simulink model "CCMPowerMode"  (R2022b)
settings: Solver=FixedStepDiscrete  StartTime=0.0  StopTime=10.0  FixedStep=0.05  RelTol=1e-3
model workspace (MAT data in the model file):
  NvMBlockPowerModeCntData = Simulink.Signal
  NvMBlockPowerModeCntData.DataType = 'UInt8'
  NvMBlockPowerModeCntData.Dimensions = [14 1]
  NvMBlockPowerModeCntData.DimensionsMode = 'auto'
  NvMBlockPowerModeCntData.Complexity = 'real'
  NvMBlockPowerModeCntData.SampleTime = -1
  NvMBlockPowerModeCntData.SamplingMode = 'auto'
  NvMBlockPowerModeCntData.InitialValue = 'NvMBlockPowerModeCntDataInit'
  NvMBlockPowerModeCntDataInit = Simulink.Parameter
  NvMBlockPowerModeCntDataInit.Value = [0; 0; 0; 0; 0; 0; 0; 0; 0; 0; 0; 0; 0; 0]
  NvMBlockPowerModeCntDataInit.DataType = 'UInt8'
  NvMBlockPowerModeCntDataInit.Complexity = 'real'
  NvMBlockPowerModeCntDataInit.Dimensions = [14 1]
undefined symbols (not defined in the file or in its folder): HFAChlType, NvMBlockId, P_Std_ReturnType, VGAChlType
settings Model: Creator=blue.ge  LastModifiedBy=blue.ge
settings DataIOCC: SaveFormat=StructureWithTime
settings OptimizationCC: DefaultParameterBehavior=Inlined  EnhancedBackFolding=on  PassReuseOutputArgsAs=Individual arguments  ZeroExternalMemoryAtStartup=off  ZeroInternalMemoryAtStartup=off  OptimizeBlockOrder=speed  DifferentSizesBufferReuse=on  OptimizationCustomize=off  EfficientTunableParamExpr=on
settings DebuggingCC: ParameterTunabilityLossMsg=error
settings ModelReferenceCC: ModelReferenceNumInstancesAllowed=Single
settings SFSimCC: MATLABDynamicMemAlloc=off
settings RTWCC: Description=AUTOSAR  SystemTargetFile=autosar.tlc  GenCodeOnly=on  TemplateMakefile=ert_default_tmf
settings CodeAppCC: ForceParamTrailComments=on  IgnoreCustomStorageClasses=off  OperatorAnnotations=on  SimulinkDataObjDesc=on  SFDataObjDesc=on  InsertBlockDesc=on
settings STFCustomTargetCC: TargetLangStandard=C99 (ISO)  MultiwordLength=256  ParMdlRefBuildCompliant=on  CompOptLevelCompliant=on  IncludeMdlTerminateFcn=off  CombineOutputUpdateFcns=on  SuppressErrorStatus=on  ERTCustomFileBanners=on  SupportNonFinite=off  SupportComplex=off  SupportContinuousTime=off  SupportNonInlinedSFcns=off  RemoveResetFunc=on  MATLABClassNameForMDSCustomization=Simulink.SoftwareTarget.ERTCustomization  AutosarCompliant=on  UseToolchainInfoCompliant=on  LookupTableObjectStructAxisOrder=2,1,3,4,...  LUTObjectStructOrderEvenSpacing=Size,Table,Breakpoints  SystemTargetFile=autosar.tlc
settings ERTTargetCC: TargetLangStandard=C99 (ISO)  ConcurrentExecutionCompliant=off  IncludeMdlTerminateFcn=off  SuppressErrorStatus=on  SupportNonFinite=off  SupportComplex=off  ModelStepFunctionPrototypeControlCompliant=off  CPPClassGenCompliant=off  AutosarCompliant=on  LookupTableObjectStructAxisOrder=2,1,3,4,...  LUTObjectStructOrderEvenSpacing=Size,Table,Breakpoints  InlinedParameterPlacement=NonHierarchical  ERTCustomFileTemplate=example_file_process.tlc

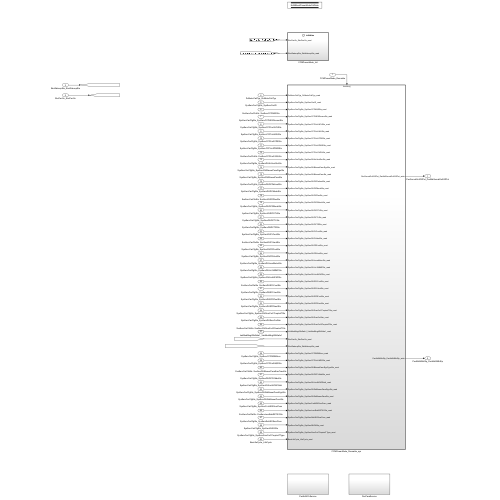
[thumbnail: Simulink's stored preview of the top-level diagram]
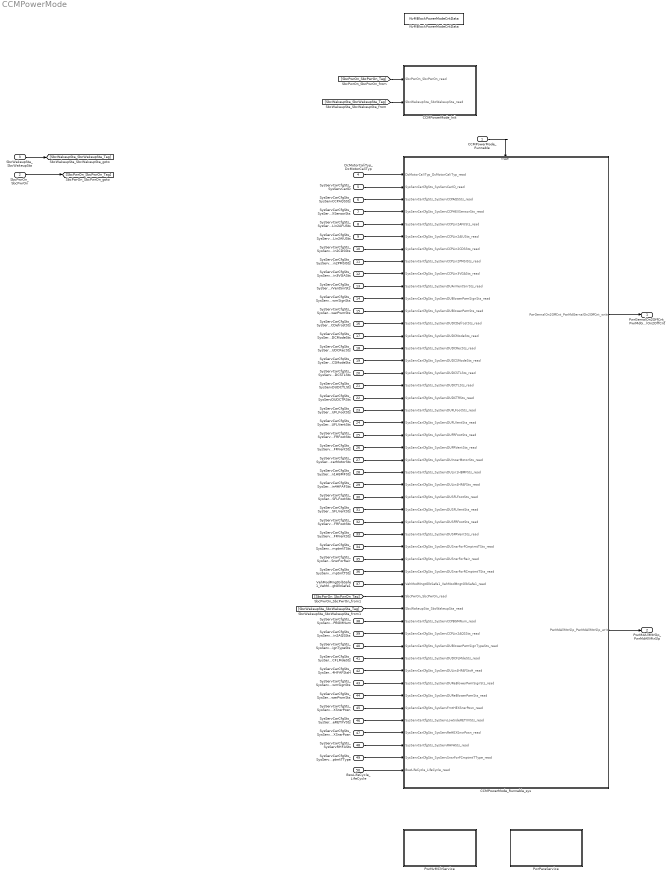
== system CCMPowerMode ==
  CCMPowerMode_Runnable : Inport  SampleTime=0.05  OutputFunctionCall=on
  SbcPwrOn_SbcPwrOn : Inport  Port=2  OutDataTypeStr=PwrOnff  PortDimensions=1
  SbcWakeupSta_SbcWakeupSta : Inport  Port=3  OutDataTypeStr=Enum: SbcWakeupSta  PortDimensions=1
  DcMotorCaliTyp_DcMotorCaliTyp : Inport  Port=4  OutDataTypeStr=UInt8  PortDimensions=1
  SysServCarCfgSts_SysServCarID : Inport  Port=5  OutDataTypeStr=Enum: SysServCarID  PortDimensions=1
  SysServCarCfgSts_SysServCCPAQSSts : Inport  Port=6  OutDataTypeStr=UInt8  PortDimensions=1
  SysServCarCfgSts_SysServCCPHEXSensorSts : Inport  Port=7  OutDataTypeStr=UInt8  PortDimensions=1
  SysServCarCfgSts_SysServCCPLin2AFUSts : Inport  Port=8  OutDataTypeStr=Boolean  PortDimensions=1
  SysServCarCfgSts_SysServCCPLin2AIUSts : Inport  Port=9  OutDataTypeStr=UInt8  PortDimensions=1
  SysServCarCfgSts_SysServCCPLin2CDSSts : Inport  Port=10  OutDataTypeStr=Boolean  PortDimensions=1
  SysServCarCfgSts_SysServCCPLin2PMSISts : Inport  Port=11  OutDataTypeStr=Boolean  PortDimensions=1
  SysServCarCfgSts_SysServCCPLin3VGASts : Inport  Port=12  OutDataTypeStr=Boolean  PortDimensions=1
  SysServCarCfgSts_SysServDUAirVentSnrSts : Inport  Port=13  OutDataTypeStr=Boolean  PortDimensions=1
  SysServCarCfgSts_SysServDUBlowerPwmSignSts : Inport  Port=14  OutDataTypeStr=Boolean  PortDimensions=1
  SysServCarCfgSts_SysServDUBlowerPwmSts : Inport  Port=15  OutDataTypeStr=Boolean  PortDimensions=1
  SysServCarCfgSts_SysServDUDCDefrostSts : Inport  Port=16  OutDataTypeStr=UInt8  PortDimensions=1
  SysServCarCfgSts_SysServDUDCModeSts : Inport  Port=17  OutDataTypeStr=UInt8  PortDimensions=1
  SysServCarCfgSts_SysServDUDCRecSts : Inport  Port=18  OutDataTypeStr=UInt8  PortDimensions=1
  SysServCarCfgSts_SysServDUDCSModeSts : Inport  Port=19  OutDataTypeStr=UInt8  PortDimensions=1
  SysServCarCfgSts_SysServDUDCSTLSts : Inport  Port=20  OutDataTypeStr=UInt8  PortDimensions=1
  SysServCarCfgSts_SysServDUDCTLSts : Inport  Port=21  OutDataTypeStr=UInt8  PortDimensions=1
  SysServCarCfgSts_SysServDUDCTRSts : Inport  Port=22  OutDataTypeStr=UInt8  PortDimensions=1
  SysServCarCfgSts_SysServDUFLFootSts : Inport  Port=23  OutDataTypeStr=UInt8  PortDimensions=1
  SysServCarCfgSts_SysServDUFLVentSts : Inport  Port=24  OutDataTypeStr=UInt8  PortDimensions=1
  SysServCarCfgSts_SysServDUFRFootSts : Inport  Port=25  OutDataTypeStr=UInt8  PortDimensions=1
  SysServCarCfgSts_SysServDUFRVentSts : Inport  Port=26  OutDataTypeStr=UInt8  PortDimensions=1
  SysServCarCfgSts_SysServDUIncarMotorSts : Inport  Port=27  OutDataTypeStr=Boolean  PortDimensions=1
  SysServCarCfgSts_SysServDULin1HBMFSts : Inport  Port=28  OutDataTypeStr=Boolean  PortDimensions=1
  SysServCarCfgSts_SysServDULin4HFAFSts : Inport  Port=29  OutDataTypeStr=UInt32  PortDimensions=1
  SysServCarCfgSts_SysServDUSFLFootSts : Inport  Port=30  OutDataTypeStr=UInt8  PortDimensions=1
  SysServCarCfgSts_SysServDUSFLVentSts : Inport  Port=31  OutDataTypeStr=UInt8  PortDimensions=1
  SysServCarCfgSts_SysServDUSFRFootSts : Inport  Port=32  OutDataTypeStr=UInt8  PortDimensions=1
  SysServCarCfgSts_SysServDUSFRVentSts : Inport  Port=33  OutDataTypeStr=UInt8  PortDimensions=1
  SysServCarCfgSts_SysServDUSnsrForFCmptmtTSts : Inport  Port=34  OutDataTypeStr=UInt8  PortDimensions=1
  SysServCarCfgSts_SysServDUSnsrForRair : Inport  Port=35  OutDataTypeStr=UInt8  PortDimensions=1
  SysServCarCfgSts_SysServDUSnsrForRCmptmtTSts : Inport  Port=36  OutDataTypeStr=UInt8  PortDimensions=1
  VehModMngtGlbSafe1_VehModMngtGlbSafe1 : Inport  Port=37  OutDataTypeStr=Bus: VehModMngtGlbSafe1  PortDimensions=1  BusOutputAsStruct=on
  SysServCarCfgSts_SysServCCPBGMNum : Inport  Port=38  OutDataTypeStr=UInt8  PortDimensions=1
  SysServCarCfgSts_SysServCCPLin2AQSSts : Inport  Port=39  OutDataTypeStr=Boolean  PortDimensions=1
  SysServCarCfgSts_SysServDUBlowerPwmSignTypeSts : Inport  Port=40  OutDataTypeStr=UInt8  PortDimensions=1
  SysServCarCfgSts_SysServDUDCFLMdeSts : Inport  Port=41  OutDataTypeStr=UInt8  PortDimensions=1
  SysServCarCfgSts_SysServDULin4HFAFStsH : Inport  Port=42  OutDataTypeStr=UInt32  PortDimensions=1
  SysServCarCfgSts_SysServDUReBlowerPwmSignSts : Inport  Port=43  OutDataTypeStr=UInt8  PortDimensions=1
  SysServCarCfgSts_SysServDUReBlowerPwmSts : Inport  Port=44  OutDataTypeStr=UInt8  PortDimensions=1
  SysServCarCfgSts_SysServFrntHEXSnsrPosn : Inport  Port=45  OutDataTypeStr=UInt8  PortDimensions=1
  SysServCarCfgSts_SysServLowSideRETXVSts : Inport  Port=46  OutDataTypeStr=UInt8  PortDimensions=1
  SysServCarCfgSts_SysServReHEXSnsrPosn : Inport  Port=47  OutDataTypeStr=UInt8  PortDimensions=1
  SysServCarCfgSts_SysServRHFASts : Inport  Port=48  OutDataTypeStr=UInt8  PortDimensions=1
  SysServCarCfgSts_SysServSnsrForFCmptmtTType : Inport  Port=49  OutDataTypeStr=UInt8  PortDimensions=1
  BswLifeCycle_LifeCycle : Inport  Port=50  OutDataTypeStr=Enum: CCMLifeCycleModeGroup  PortDimensions=1
  CCMPowerMode_Init : Subsystem
  CCMPowerMode_Runnable_sys : Subsystem
  NvMBlockPowerModeCntData : DataStoreMemory  DataStoreName=NvMBlockPowerModeCntData  InitialValue=NvMBlockPowerModeCntDataInit  ShowAdditionalParam=off  Dimensions=14  StateMustResolveToSignalObject=on
  PwrNvMClrService : Subsystem
  PwrParaService : Subsystem
  PwrGernalOn2OffCnt_PwrMdGernalOn2OffCnt : Outport  OutDataTypeStr=On2OffCnt  PortDimensions=1
  PwrMdAllMtrSlp_PwrMdAllMtrSlp : Outport  Port=2  OutDataTypeStr=Enum: PwrMdAllMtrSlp  PortDimensions=1
  wires:
  DcMotorCaliTyp_DcMotorCaliTyp/1 -> CCMPowerMode_Runnable_sys/1(DcMotorCaliTyp_DcMotorCaliTyp_read)
  SysServCarCfgSts_SysServCarID/1 -> CCMPowerMode_Runnable_sys/2(SysServCarCfgSts_SysServCarID_read)
  SysServCarCfgSts_SysServCCPAQSSts/1 -> CCMPowerMode_Runnable_sys/3(SysServCarCfgSts_SysServCCPAQSSts_read)
  SysServCarCfgSts_SysServCCPHEXSensorSts/1 -> CCMPowerMode_Runnable_sys/4(SysServCarCfgSts_SysServCCPHEXSensorSts_read)
  SysServCarCfgSts_SysServCCPLin2AFUSts/1 -> CCMPowerMode_Runnable_sys/5(SysServCarCfgSts_SysServCCPLin2AFUSts_read)
  SysServCarCfgSts_SysServCCPLin2AIUSts/1 -> CCMPowerMode_Runnable_sys/6(SysServCarCfgSts_SysServCCPLin2AIUSts_read)
  SysServCarCfgSts_SysServCCPLin2CDSSts/1 -> CCMPowerMode_Runnable_sys/7(SysServCarCfgSts_SysServCCPLin2CDSSts_read)
  SysServCarCfgSts_SysServCCPLin2PMSISts/1 -> CCMPowerMode_Runnable_sys/8(SysServCarCfgSts_SysServCCPLin2PMSISts_read)
  SysServCarCfgSts_SysServCCPLin3VGASts/1 -> CCMPowerMode_Runnable_sys/9(SysServCarCfgSts_SysServCCPLin3VGASts_read)
  SysServCarCfgSts_SysServDUAirVentSnrSts/1 -> CCMPowerMode_Runnable_sys/10(SysServCarCfgSts_SysServDUAirVentSnrSts_read)
  SysServCarCfgSts_SysServDUBlowerPwmSignSts/1 -> CCMPowerMode_Runnable_sys/11(SysServCarCfgSts_SysServDUBlowerPwmSignSts_read)
  SysServCarCfgSts_SysServDUBlowerPwmSts/1 -> CCMPowerMode_Runnable_sys/12(SysServCarCfgSts_SysServDUBlowerPwmSts_read)
  SysServCarCfgSts_SysServDUDCDefrostSts/1 -> CCMPowerMode_Runnable_sys/13(SysServCarCfgSts_SysServDUDCDefrostSts_read)
  SysServCarCfgSts_SysServDUDCModeSts/1 -> CCMPowerMode_Runnable_sys/14(SysServCarCfgSts_SysServDUDCModeSts_read)
  SysServCarCfgSts_SysServDUDCRecSts/1 -> CCMPowerMode_Runnable_sys/15(SysServCarCfgSts_SysServDUDCRecSts_read)
  SysServCarCfgSts_SysServDUDCSModeSts/1 -> CCMPowerMode_Runnable_sys/16(SysServCarCfgSts_SysServDUDCSModeSts_read)
  SysServCarCfgSts_SysServDUDCSTLSts/1 -> CCMPowerMode_Runnable_sys/17(SysServCarCfgSts_SysServDUDCSTLSts_read)
  SysServCarCfgSts_SysServDUDCTLSts/1 -> CCMPowerMode_Runnable_sys/18(SysServCarCfgSts_SysServDUDCTLSts_read)
  SysServCarCfgSts_SysServDUDCTRSts/1 -> CCMPowerMode_Runnable_sys/19(SysServCarCfgSts_SysServDUDCTRSts_read)
  SysServCarCfgSts_SysServDUFLFootSts/1 -> CCMPowerMode_Runnable_sys/20(SysServCarCfgSts_SysServDUFLFootSts_read)
  SysServCarCfgSts_SysServDUFLVentSts/1 -> CCMPowerMode_Runnable_sys/21(SysServCarCfgSts_SysServDUFLVentSts_read)
  SysServCarCfgSts_SysServDUFRFootSts/1 -> CCMPowerMode_Runnable_sys/22(SysServCarCfgSts_SysServDUFRFootSts_read)
  SysServCarCfgSts_SysServDUFRVentSts/1 -> CCMPowerMode_Runnable_sys/23(SysServCarCfgSts_SysServDUFRVentSts_read)
  SysServCarCfgSts_SysServDUIncarMotorSts/1 -> CCMPowerMode_Runnable_sys/24(SysServCarCfgSts_SysServDUIncarMotorSts_read)
  SysServCarCfgSts_SysServDULin1HBMFSts/1 -> CCMPowerMode_Runnable_sys/25(SysServCarCfgSts_SysServDULin1HBMFSts_read)
  SysServCarCfgSts_SysServDULin4HFAFSts/1 -> CCMPowerMode_Runnable_sys/26(SysServCarCfgSts_SysServDULin4HFAFSts_read)
  SysServCarCfgSts_SysServDUSFLFootSts/1 -> CCMPowerMode_Runnable_sys/27(SysServCarCfgSts_SysServDUSFLFootSts_read)
  SysServCarCfgSts_SysServDUSFLVentSts/1 -> CCMPowerMode_Runnable_sys/28(SysServCarCfgSts_SysServDUSFLVentSts_read)
  SysServCarCfgSts_SysServDUSFRFootSts/1 -> CCMPowerMode_Runnable_sys/29(SysServCarCfgSts_SysServDUSFRFootSts_read)
  SysServCarCfgSts_SysServDUSFRVentSts/1 -> CCMPowerMode_Runnable_sys/30(SysServCarCfgSts_SysServDUSFRVentSts_read)
  SysServCarCfgSts_SysServDUSnsrForFCmptmtTSts/1 -> CCMPowerMode_Runnable_sys/31(SysServCarCfgSts_SysServDUSnsrForFCmptmtTSts_read)
  SysServCarCfgSts_SysServDUSnsrForRair/1 -> CCMPowerMode_Runnable_sys/32(SysServCarCfgSts_SysServDUSnsrForRair_read)
  SysServCarCfgSts_SysServDUSnsrForRCmptmtTSts/1 -> CCMPowerMode_Runnable_sys/33(SysServCarCfgSts_SysServDUSnsrForRCmptmtTSts_read)
  VehModMngtGlbSafe1_VehModMngtGlbSafe1/1 -> CCMPowerMode_Runnable_sys/34(VehModMngtGlbSafe1_VehModMngtGlbSafe1_read)
  SysServCarCfgSts_SysServCCPBGMNum/1 -> CCMPowerMode_Runnable_sys/37(SysServCarCfgSts_SysServCCPBGMNum_read)
  SysServCarCfgSts_SysServCCPLin2AQSSts/1 -> CCMPowerMode_Runnable_sys/38(SysServCarCfgSts_SysServCCPLin2AQSSts_read)
  SysServCarCfgSts_SysServDUBlowerPwmSignTypeSts/1 -> CCMPowerMode_Runnable_sys/39(SysServCarCfgSts_SysServDUBlowerPwmSignTypeSts_read)
  SysServCarCfgSts_SysServDUDCFLMdeSts/1 -> CCMPowerMode_Runnable_sys/40(SysServCarCfgSts_SysServDUDCFLMdeSts_read)
  SysServCarCfgSts_SysServDULin4HFAFStsH/1 -> CCMPowerMode_Runnable_sys/41(SysServCarCfgSts_SysServDULin4HFAFStsH_read)
  SysServCarCfgSts_SysServDUReBlowerPwmSignSts/1 -> CCMPowerMode_Runnable_sys/42(SysServCarCfgSts_SysServDUReBlowerPwmSignSts_read)
  SysServCarCfgSts_SysServDUReBlowerPwmSts/1 -> CCMPowerMode_Runnable_sys/43(SysServCarCfgSts_SysServDUReBlowerPwmSts_read)
  SysServCarCfgSts_SysServFrntHEXSnsrPosn/1 -> CCMPowerMode_Runnable_sys/44(SysServCarCfgSts_SysServFrntHEXSnsrPosn_read)
  SysServCarCfgSts_SysServLowSideRETXVSts/1 -> CCMPowerMode_Runnable_sys/45(SysServCarCfgSts_SysServLowSideRETXVSts_read)
  SysServCarCfgSts_SysServReHEXSnsrPosn/1 -> CCMPowerMode_Runnable_sys/46(SysServCarCfgSts_SysServReHEXSnsrPosn_read)
  SysServCarCfgSts_SysServRHFASts/1 -> CCMPowerMode_Runnable_sys/47(SysServCarCfgSts_SysServRHFASts_read)
  SysServCarCfgSts_SysServSnsrForFCmptmtTType/1 -> CCMPowerMode_Runnable_sys/48(SysServCarCfgSts_SysServSnsrForFCmptmtTType_read)
  CCMPowerMode_Runnable_sys/1(PwrGernalOn2OffCnt_PwrMdGernalOn2OffCnt_write) -> PwrGernalOn2OffCnt_PwrMdGernalOn2OffCnt/1
  CCMPowerMode_Runnable_sys/2(PwrMdAllMtrSlp_PwrMdAllMtrSlp_write) -> PwrMdAllMtrSlp_PwrMdAllMtrSlp/1
  BswLifeCycle_LifeCycle/1 -> CCMPowerMode_Runnable_sys/49(BswLifeCycle_LifeCycle_read)
  SbcWakeupSta_SbcWakeupSta/1 -> [SbcWakeupSta_SbcWakeupSta_Tag]
  [SbcWakeupSta_SbcWakeupSta_Tag] -> CCMPowerMode_Init/2(SbcWakeupSta_SbcWakeupSta_read)
  [SbcWakeupSta_SbcWakeupSta_Tag] -> CCMPowerMode_Runnable_sys/36(SbcWakeupSta_SbcWakeupSta_read)
  SbcPwrOn_SbcPwrOn/1 -> [SbcPwrOn_SbcPwrOn_Tag]
  [SbcPwrOn_SbcPwrOn_Tag] -> CCMPowerMode_Init/1(SbcPwrOn_SbcPwrOn_read)
  [SbcPwrOn_SbcPwrOn_Tag] -> CCMPowerMode_Runnable_sys/35(SbcPwrOn_SbcPwrOn_read)
  CCMPowerMode_Runnable/1 -> CCMPowerMode_Runnable_sys/Trigger

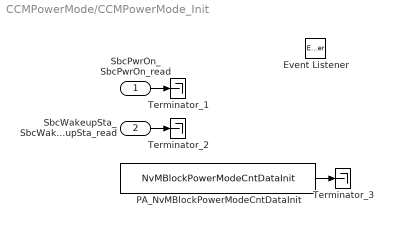
== system CCMPowerMode/CCMPowerMode_Init ==
  SbcPwrOn_SbcPwrOn_read : Inport
  SbcWakeupSta_SbcWakeupSta_read : Inport  Port=2
  Event Listener : EventListener  EventName=reset
  PA_NvMBlockPowerModeCntDataInit : Constant  Value=NvMBlockPowerModeCntDataInit
  Terminator_1 : Terminator
  Terminator_2 : Terminator
  Terminator_3 : Terminator
  wires:
  SbcPwrOn_SbcPwrOn_read/1 -> Terminator_1/1
  SbcWakeupSta_SbcWakeupSta_read/1 -> Terminator_2/1
  PA_NvMBlockPowerModeCntDataInit/1 -> Terminator_3/1

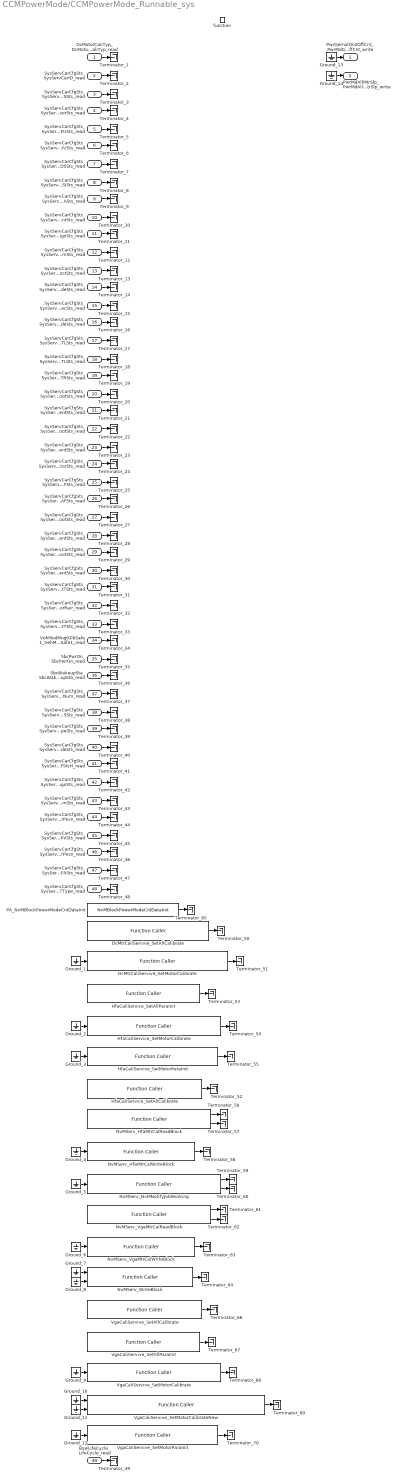
== system CCMPowerMode/CCMPowerMode_Runnable_sys ==
  DcMotorCaliTyp_DcMotorCaliTyp_read : Inport
  SysServCarCfgSts_SysServCarID_read : Inport  Port=2
  SysServCarCfgSts_SysServCCPAQSSts_read : Inport  Port=3
  SysServCarCfgSts_SysServCCPHEXSensorSts_read : Inport  Port=4
  SysServCarCfgSts_SysServCCPLin2AFUSts_read : Inport  Port=5
  SysServCarCfgSts_SysServCCPLin2AIUSts_read : Inport  Port=6
  SysServCarCfgSts_SysServCCPLin2CDSSts_read : Inport  Port=7
  SysServCarCfgSts_SysServCCPLin2PMSISts_read : Inport  Port=8
  SysServCarCfgSts_SysServCCPLin3VGASts_read : Inport  Port=9
  SysServCarCfgSts_SysServDUAirVentSnrSts_read : Inport  Port=10
  SysServCarCfgSts_SysServDUBlowerPwmSignSts_read : Inport  Port=11
  SysServCarCfgSts_SysServDUBlowerPwmSts_read : Inport  Port=12
  SysServCarCfgSts_SysServDUDCDefrostSts_read : Inport  Port=13
  SysServCarCfgSts_SysServDUDCModeSts_read : Inport  Port=14
  SysServCarCfgSts_SysServDUDCRecSts_read : Inport  Port=15
  SysServCarCfgSts_SysServDUDCSModeSts_read : Inport  Port=16
  SysServCarCfgSts_SysServDUDCSTLSts_read : Inport  Port=17
  SysServCarCfgSts_SysServDUDCTLSts_read : Inport  Port=18
  SysServCarCfgSts_SysServDUDCTRSts_read : Inport  Port=19
  SysServCarCfgSts_SysServDUFLFootSts_read : Inport  Port=20
  SysServCarCfgSts_SysServDUFLVentSts_read : Inport  Port=21
  SysServCarCfgSts_SysServDUFRFootSts_read : Inport  Port=22
  SysServCarCfgSts_SysServDUFRVentSts_read : Inport  Port=23
  SysServCarCfgSts_SysServDUIncarMotorSts_read : Inport  Port=24
  SysServCarCfgSts_SysServDULin1HBMFSts_read : Inport  Port=25
  SysServCarCfgSts_SysServDULin4HFAFSts_read : Inport  Port=26
  SysServCarCfgSts_SysServDUSFLFootSts_read : Inport  Port=27
  SysServCarCfgSts_SysServDUSFLVentSts_read : Inport  Port=28
  SysServCarCfgSts_SysServDUSFRFootSts_read : Inport  Port=29
  SysServCarCfgSts_SysServDUSFRVentSts_read : Inport  Port=30
  SysServCarCfgSts_SysServDUSnsrForFCmptmtTSts_read : Inport  Port=31
  SysServCarCfgSts_SysServDUSnsrForRair_read : Inport  Port=32
  SysServCarCfgSts_SysServDUSnsrForRCmptmtTSts_read : Inport  Port=33
  VehModMngtGlbSafe1_VehModMngtGlbSafe1_read : Inport  Port=34
  SbcPwrOn_SbcPwrOn_read : Inport  Port=35
  SbcWakeupSta_SbcWakeupSta_read : Inport  Port=36
  SysServCarCfgSts_SysServCCPBGMNum_read : Inport  Port=37
  SysServCarCfgSts_SysServCCPLin2AQSSts_read : Inport  Port=38
  SysServCarCfgSts_SysServDUBlowerPwmSignTypeSts_read : Inport  Port=39
  SysServCarCfgSts_SysServDUDCFLMdeSts_read : Inport  Port=40
  SysServCarCfgSts_SysServDULin4HFAFStsH_read : Inport  Port=41
  SysServCarCfgSts_SysServDUReBlowerPwmSignSts_read : Inport  Port=42
  SysServCarCfgSts_SysServDUReBlowerPwmSts_read : Inport  Port=43
  SysServCarCfgSts_SysServFrntHEXSnsrPosn_read : Inport  Port=44
  SysServCarCfgSts_SysServLowSideRETXVSts_read : Inport  Port=45
  SysServCarCfgSts_SysServReHEXSnsrPosn_read : Inport  Port=46
  SysServCarCfgSts_SysServRHFASts_read : Inport  Port=47
  SysServCarCfgSts_SysServSnsrForFCmptmtTType_read : Inport  Port=48
  BswLifeCycle_LifeCycle_read : Inport  Port=49
  function : TriggerPort  TriggerType=function-call  VariantControl=(inherit)  SampleTimeType=periodic  SampleTime=-1
  DcMtrCaliServive_SetAllCalibrate : FunctionCaller  FunctionPrototype=ERR = DcMtrCaliServive_SetAllCalibrate()  OutputArgumentSpecifications=P_Std_ReturnType
  DcMtrCaliServive_SetMotorCalibrate : FunctionCaller  FunctionPrototype=ERR = DcMtrCaliServive_SetMotorCalibrate(Channel)  InputArgumentSpecifications=uint8(1)  OutputArgumentSpecifications=P_Std_ReturnType
  Ground_{1..14} (14 alike) : Ground
  HfaCaliService_SetAllCalibrate : FunctionCaller  FunctionPrototype=ERR = HfaCaliService_SetAllCalibrate()  OutputArgumentSpecifications=P_Std_ReturnType
  HfaCaliService_SetAllParaInit : FunctionCaller  FunctionPrototype=ERR = HfaCaliService_SetAllParaInit()  OutputArgumentSpecifications=P_Std_ReturnType
  HfaCaliService_SetMotorCalibrate : FunctionCaller  FunctionPrototype=ERR = HfaCaliService_SetMotorCalibrate(Channel)  InputArgumentSpecifications=HFAChlType(0)  OutputArgumentSpecifications=P_Std_ReturnType
  HfaCaliService_SetMotorParaInit : FunctionCaller  FunctionPrototype=ERR = HfaCaliService_SetMotorParaInit(Channel)  InputArgumentSpecifications=HFAChlType(0)  OutputArgumentSpecifications=P_Std_ReturnType
  NvMServ_HfaMtrCalReadBlock : FunctionCaller  FunctionPrototype=[Data,ERR] = NvMServ_HfaMtrCalReadBlock()  OutputArgumentSpecifications=uint8([1]), P_Std_ReturnType
  NvMServ_HfaMtrCalWriteBlock : FunctionCaller  FunctionPrototype=ERR = NvMServ_HfaMtrCalWriteBlock(Data)  InputArgumentSpecifications=uint8([1])  OutputArgumentSpecifications=P_Std_ReturnType
  NvMServ_NvMNotifyJobWorking : FunctionCaller  FunctionPrototype=[Status,ERR] = NvMServ_NvMNotifyJobWorking(BlockId)  InputArgumentSpecifications=NvMBlockId(0)  OutputArgumentSpecifications=uint8(1), P_Std_ReturnType
  NvMServ_VgaMtrCalReadBlock : FunctionCaller  FunctionPrototype=[Data,ERR] = NvMServ_VgaMtrCalReadBlock()  OutputArgumentSpecifications=uint8([1]), P_Std_ReturnType
  NvMServ_VgaMtrCalWriteBlock : FunctionCaller  FunctionPrototype=ERR = NvMServ_VgaMtrCalWriteBlock(Data)  InputArgumentSpecifications=uint8([1])  OutputArgumentSpecifications=P_Std_ReturnType
  NvMServ_WriteBlock : FunctionCaller  FunctionPrototype=ERR = NvMServ_WriteBlock(BlockId,Data)  InputArgumentSpecifications=NvMBlockId(0), uint8([1])  OutputArgumentSpecifications=P_Std_ReturnType
  PA_NvMBlockPowerModeCntDataInit : Constant  Value=NvMBlockPowerModeCntDataInit
  Terminator_{1..70} (70 alike) : Terminator
  VgaCaliServive_SetAllCalibrate : FunctionCaller  FunctionPrototype=ERR = VgaCaliServive_SetAllCalibrate()  OutputArgumentSpecifications=P_Std_ReturnType
  VgaCaliServive_SetAllParaInit : FunctionCaller  FunctionPrototype=ERR = VgaCaliServive_SetAllParaInit()  OutputArgumentSpecifications=P_Std_ReturnType
  VgaCaliServive_SetMotorCalibrate : FunctionCaller  FunctionPrototype=ERR = VgaCaliServive_SetMotorCalibrate(Channel)  InputArgumentSpecifications=VGAChlType(0)  OutputArgumentSpecifications=P_Std_ReturnType
  VgaCaliServive_SetMotorCalibrateNew : FunctionCaller  FunctionPrototype=ERR = VgaCaliServive_SetMotorCalibrateNew(Channel,LearnType)  InputArgumentSpecifications=VGAChlType(0), uint8(1)  OutputArgumentSpecifications=P_Std_ReturnType
  VgaCaliServive_SetMotorParaInit : FunctionCaller  FunctionPrototype=ERR = VgaCaliServive_SetMotorParaInit(Channel)  InputArgumentSpecifications=VGAChlType(0)  OutputArgumentSpecifications=P_Std_ReturnType
  PwrGernalOn2OffCnt_PwrMdGernalOn2OffCnt_write : Outport
      settings: EnsureOutportIsVirtual=on
  PwrMdAllMtrSlp_PwrMdAllMtrSlp_write : Outport  Port=2
      settings: EnsureOutportIsVirtual=on
  wires:
  DcMotorCaliTyp_DcMotorCaliTyp_read/1 -> Terminator_1/1
  SysServCarCfgSts_SysServCarID_read/1 -> Terminator_2/1
  SysServCarCfgSts_SysServCCPAQSSts_read/1 -> Terminator_3/1
  SysServCarCfgSts_SysServCCPHEXSensorSts_read/1 -> Terminator_4/1
  SysServCarCfgSts_SysServCCPLin2AFUSts_read/1 -> Terminator_5/1
  SysServCarCfgSts_SysServCCPLin2AIUSts_read/1 -> Terminator_6/1
  SysServCarCfgSts_SysServCCPLin2CDSSts_read/1 -> Terminator_7/1
  SysServCarCfgSts_SysServCCPLin2PMSISts_read/1 -> Terminator_8/1
  SysServCarCfgSts_SysServCCPLin3VGASts_read/1 -> Terminator_9/1
  SysServCarCfgSts_SysServDUAirVentSnrSts_read/1 -> Terminator_10/1
  SysServCarCfgSts_SysServDUBlowerPwmSignSts_read/1 -> Terminator_11/1
  SysServCarCfgSts_SysServDUBlowerPwmSts_read/1 -> Terminator_12/1
  SysServCarCfgSts_SysServDUDCDefrostSts_read/1 -> Terminator_13/1
  SysServCarCfgSts_SysServDUDCModeSts_read/1 -> Terminator_14/1
  SysServCarCfgSts_SysServDUDCRecSts_read/1 -> Terminator_15/1
  SysServCarCfgSts_SysServDUDCSModeSts_read/1 -> Terminator_16/1
  SysServCarCfgSts_SysServDUDCSTLSts_read/1 -> Terminator_17/1
  SysServCarCfgSts_SysServDUDCTLSts_read/1 -> Terminator_18/1
  SysServCarCfgSts_SysServDUDCTRSts_read/1 -> Terminator_19/1
  SysServCarCfgSts_SysServDUFLFootSts_read/1 -> Terminator_20/1
  SysServCarCfgSts_SysServDUFLVentSts_read/1 -> Terminator_21/1
  SysServCarCfgSts_SysServDUFRFootSts_read/1 -> Terminator_22/1
  SysServCarCfgSts_SysServDUFRVentSts_read/1 -> Terminator_23/1
  SysServCarCfgSts_SysServDUIncarMotorSts_read/1 -> Terminator_24/1
  SysServCarCfgSts_SysServDULin1HBMFSts_read/1 -> Terminator_25/1
  SysServCarCfgSts_SysServDULin4HFAFSts_read/1 -> Terminator_26/1
  SysServCarCfgSts_SysServDUSFLFootSts_read/1 -> Terminator_27/1
  SysServCarCfgSts_SysServDUSFLVentSts_read/1 -> Terminator_28/1
  SysServCarCfgSts_SysServDUSFRFootSts_read/1 -> Terminator_29/1
  SysServCarCfgSts_SysServDUSFRVentSts_read/1 -> Terminator_30/1
  SysServCarCfgSts_SysServDUSnsrForFCmptmtTSts_read/1 -> Terminator_31/1
  SysServCarCfgSts_SysServDUSnsrForRair_read/1 -> Terminator_32/1
  SysServCarCfgSts_SysServDUSnsrForRCmptmtTSts_read/1 -> Terminator_33/1
  VehModMngtGlbSafe1_VehModMngtGlbSafe1_read/1 -> Terminator_34/1
  SbcPwrOn_SbcPwrOn_read/1 -> Terminator_35/1
  SbcWakeupSta_SbcWakeupSta_read/1 -> Terminator_36/1
  SysServCarCfgSts_SysServCCPBGMNum_read/1 -> Terminator_37/1
  SysServCarCfgSts_SysServCCPLin2AQSSts_read/1 -> Terminator_38/1
  SysServCarCfgSts_SysServDUBlowerPwmSignTypeSts_read/1 -> Terminator_39/1
  SysServCarCfgSts_SysServDUDCFLMdeSts_read/1 -> Terminator_40/1
  SysServCarCfgSts_SysServDULin4HFAFStsH_read/1 -> Terminator_41/1
  SysServCarCfgSts_SysServDUReBlowerPwmSignSts_read/1 -> Terminator_42/1
  SysServCarCfgSts_SysServDUReBlowerPwmSts_read/1 -> Terminator_43/1
  SysServCarCfgSts_SysServFrntHEXSnsrPosn_read/1 -> Terminator_44/1
  SysServCarCfgSts_SysServLowSideRETXVSts_read/1 -> Terminator_45/1
  SysServCarCfgSts_SysServReHEXSnsrPosn_read/1 -> Terminator_46/1
  SysServCarCfgSts_SysServRHFASts_read/1 -> Terminator_47/1
  SysServCarCfgSts_SysServSnsrForFCmptmtTType_read/1 -> Terminator_48/1
  BswLifeCycle_LifeCycle_read/1 -> Terminator_49/1
  DcMtrCaliServive_SetAllCalibrate/1 -> Terminator_50/1
  Ground_1/1 -> DcMtrCaliServive_SetMotorCalibrate/1
  DcMtrCaliServive_SetMotorCalibrate/1 -> Terminator_51/1
  HfaCaliService_SetAllCalibrate/1 -> Terminator_52/1
  HfaCaliService_SetAllParaInit/1 -> Terminator_53/1
  Ground_2/1 -> HfaCaliService_SetMotorCalibrate/1
  HfaCaliService_SetMotorCalibrate/1 -> Terminator_54/1
  Ground_3/1 -> HfaCaliService_SetMotorParaInit/1
  HfaCaliService_SetMotorParaInit/1 -> Terminator_55/1
  NvMServ_HfaMtrCalReadBlock/1 -> Terminator_56/1
  NvMServ_HfaMtrCalReadBlock/2 -> Terminator_57/1
  Ground_4/1 -> NvMServ_HfaMtrCalWriteBlock/1
  NvMServ_HfaMtrCalWriteBlock/1 -> Terminator_58/1
  Ground_5/1 -> NvMServ_NvMNotifyJobWorking/1
  NvMServ_NvMNotifyJobWorking/1 -> Terminator_59/1
  NvMServ_NvMNotifyJobWorking/2 -> Terminator_60/1
  NvMServ_VgaMtrCalReadBlock/1 -> Terminator_61/1
  NvMServ_VgaMtrCalReadBlock/2 -> Terminator_62/1
  Ground_6/1 -> NvMServ_VgaMtrCalWriteBlock/1
  NvMServ_VgaMtrCalWriteBlock/1 -> Terminator_63/1
  Ground_7/1 -> NvMServ_WriteBlock/1
  Ground_8/1 -> NvMServ_WriteBlock/2
  NvMServ_WriteBlock/1 -> Terminator_64/1
  PA_NvMBlockPowerModeCntDataInit/1 -> Terminator_65/1
  VgaCaliServive_SetAllCalibrate/1 -> Terminator_66/1
  VgaCaliServive_SetAllParaInit/1 -> Terminator_67/1
  Ground_9/1 -> VgaCaliServive_SetMotorCalibrate/1
  VgaCaliServive_SetMotorCalibrate/1 -> Terminator_68/1
  Ground_10/1 -> VgaCaliServive_SetMotorCalibrateNew/1
  Ground_11/1 -> VgaCaliServive_SetMotorCalibrateNew/2
  VgaCaliServive_SetMotorCalibrateNew/1 -> Terminator_69/1
  Ground_12/1 -> VgaCaliServive_SetMotorParaInit/1
  VgaCaliServive_SetMotorParaInit/1 -> Terminator_70/1
  Ground_13/1 -> PwrGernalOn2OffCnt_PwrMdGernalOn2OffCnt_write/1
  Ground_14/1 -> PwrMdAllMtrSlp_PwrMdAllMtrSlp_write/1

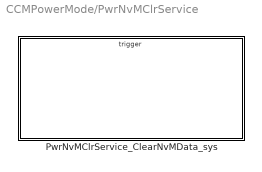
== system CCMPowerMode/PwrNvMClrService ==
  PwrNvMClrService_ClearNvMData_sys : Subsystem

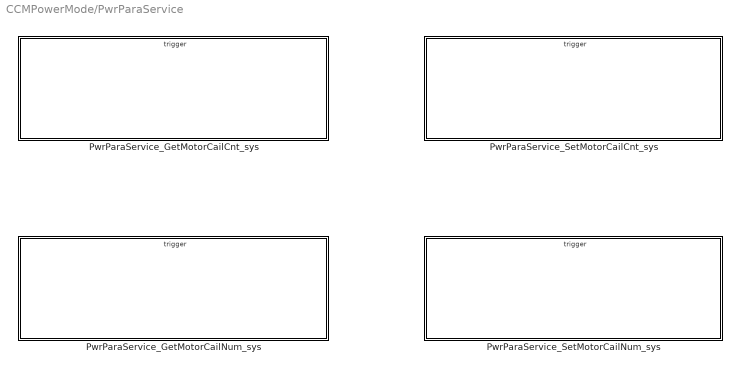
== system CCMPowerMode/PwrParaService ==
  PwrParaService_GetMotorCailCnt_sys : Subsystem
  PwrParaService_GetMotorCailNum_sys : Subsystem
  PwrParaService_SetMotorCailCnt_sys : Subsystem
  PwrParaService_SetMotorCailNum_sys : Subsystem

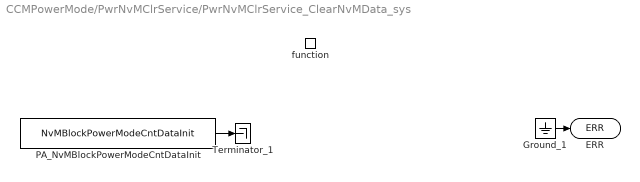
== system CCMPowerMode/PwrNvMClrService/PwrNvMClrService_ClearNvMData_sys ==
  function : TriggerPort  TriggerType=function-call  IsSimulinkFunction=on  FunctionName=PwrNvMClrService_ClearNvMData  VariantControl=(inherit)
  Ground_1 : Ground
  PA_NvMBlockPowerModeCntDataInit : Constant  Value=NvMBlockPowerModeCntDataInit
  Terminator_1 : Terminator
  ERR : ArgOut  OutDataTypeStr=Std_ReturnType  ArgumentName=ERR
  wires:
  PA_NvMBlockPowerModeCntDataInit/1 -> Terminator_1/1
  Ground_1/1 -> ERR/1

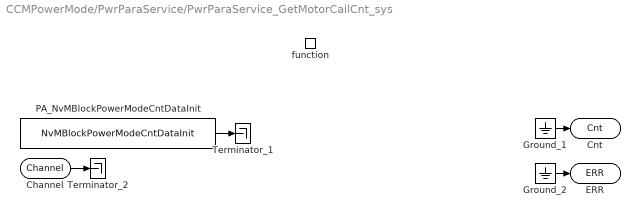
== system CCMPowerMode/PwrParaService/PwrParaService_GetMotorCailCnt_sys ==
  function : TriggerPort  TriggerType=function-call  IsSimulinkFunction=on  FunctionName=PwrParaService_GetMotorCailCnt  VariantControl=(inherit)
  Ground_1 : Ground
  Ground_2 : Ground
  PA_NvMBlockPowerModeCntDataInit : Constant  Value=NvMBlockPowerModeCntDataInit
  Terminator_1 : Terminator
  Terminator_2 : Terminator
  Channel : ArgIn  OutDataTypeStr=Enum: PowerChlType  ArgumentName=Channel
  Cnt : ArgOut  OutDataTypeStr=UInt8  ArgumentName=Cnt
  ERR : ArgOut  Port=2  OutDataTypeStr=Std_ReturnType  ArgumentName=ERR
  wires:
  PA_NvMBlockPowerModeCntDataInit/1 -> Terminator_1/1
  Channel/1 -> Terminator_2/1
  Ground_1/1 -> Cnt/1
  Ground_2/1 -> ERR/1

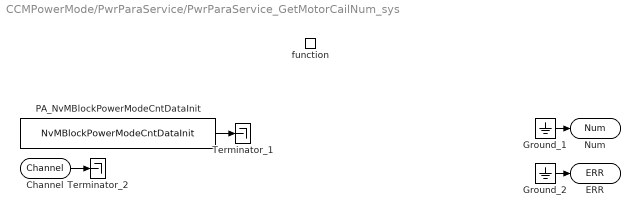
== system CCMPowerMode/PwrParaService/PwrParaService_GetMotorCailNum_sys ==
  function : TriggerPort  TriggerType=function-call  IsSimulinkFunction=on  FunctionName=PwrParaService_GetMotorCailNum  VariantControl=(inherit)
  Ground_1 : Ground
  Ground_2 : Ground
  PA_NvMBlockPowerModeCntDataInit : Constant  Value=NvMBlockPowerModeCntDataInit
  Terminator_1 : Terminator
  Terminator_2 : Terminator
  Channel : ArgIn  OutDataTypeStr=Enum: PowerChlType  ArgumentName=Channel
  Num : ArgOut  OutDataTypeStr=UInt8  ArgumentName=Num
  ERR : ArgOut  Port=2  OutDataTypeStr=Std_ReturnType  ArgumentName=ERR
  wires:
  PA_NvMBlockPowerModeCntDataInit/1 -> Terminator_1/1
  Channel/1 -> Terminator_2/1
  Ground_1/1 -> Num/1
  Ground_2/1 -> ERR/1

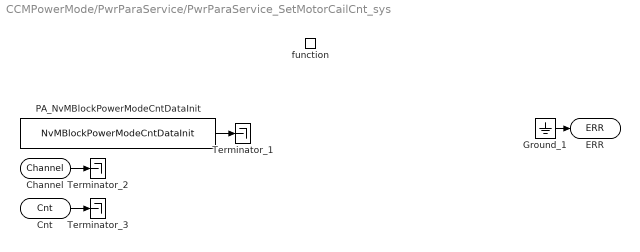
== system CCMPowerMode/PwrParaService/PwrParaService_SetMotorCailCnt_sys ==
  function : TriggerPort  TriggerType=function-call  IsSimulinkFunction=on  FunctionName=PwrParaService_SetMotorCailCnt  VariantControl=(inherit)
  Ground_1 : Ground
  PA_NvMBlockPowerModeCntDataInit : Constant  Value=NvMBlockPowerModeCntDataInit
  Terminator_1 : Terminator
  Terminator_2 : Terminator
  Terminator_3 : Terminator
  Channel : ArgIn  OutDataTypeStr=Enum: PowerChlType  ArgumentName=Channel
  Cnt : ArgIn  Port=2  OutDataTypeStr=UInt8  ArgumentName=Cnt
  ERR : ArgOut  OutDataTypeStr=Std_ReturnType  ArgumentName=ERR
  wires:
  PA_NvMBlockPowerModeCntDataInit/1 -> Terminator_1/1
  Channel/1 -> Terminator_2/1
  Cnt/1 -> Terminator_3/1
  Ground_1/1 -> ERR/1

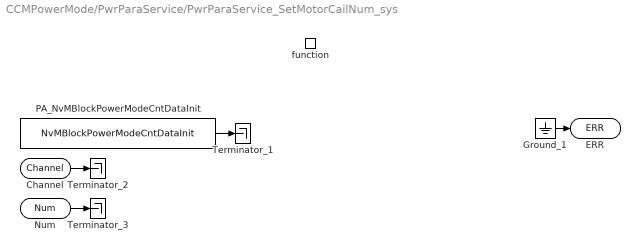
== system CCMPowerMode/PwrParaService/PwrParaService_SetMotorCailNum_sys ==
  function : TriggerPort  TriggerType=function-call  IsSimulinkFunction=on  FunctionName=PwrParaService_SetMotorCailNum  VariantControl=(inherit)
  Ground_1 : Ground
  PA_NvMBlockPowerModeCntDataInit : Constant  Value=NvMBlockPowerModeCntDataInit
  Terminator_1 : Terminator
  Terminator_2 : Terminator
  Terminator_3 : Terminator
  Channel : ArgIn  OutDataTypeStr=Enum: PowerChlType  ArgumentName=Channel
  Num : ArgIn  Port=2  OutDataTypeStr=UInt8  ArgumentName=Num
  ERR : ArgOut  OutDataTypeStr=Std_ReturnType  ArgumentName=ERR
  wires:
  PA_NvMBlockPowerModeCntDataInit/1 -> Terminator_1/1
  Channel/1 -> Terminator_2/1
  Num/1 -> Terminator_3/1
  Ground_1/1 -> ERR/1
